simulink model "TSR_Scenario_002_cs"  (Simulink 7.6)
settings: Solver=ode5  SolverMode=SingleTasking  StartTime=0.0  StopTime=inf  FixedStep=1/100  RelTol=1e-3
PreLoadFcn:
  | check_NUTS_defined;
  | 
  | assignin('base',['model_closed_' bdroot],false);
  | 
  | addpath('../includes_TSR/includes_TSR');
  | 
  | switch version('-release')
  | case {'2010a', '2010b', '2011a'}
  | addpath('../includes_TSR/ML2010b');
  | otherwise
  | addpath('../includes_TSR/ML2011b');
  | end
InitFcn:
  | PreScanExperimentInitFcn(bdroot);
  | 
  | addpath('..\includes_TSR');
StopFcn:
  | PreScanExperimentStopFcn(bdroot)
undefined symbols (not defined in the file): DiscIntNeverNeededParam, InitImageOutputDir, x_ini_VRML, y_ini_VRML, z_ini_VRML
libraries: PreScanSimulinkLibrary x79, simulink x28, PreScanUsefulBlocks x21, simulink_extras x12, simulink_need_slupdate x1
(part 3 of 36)

== system TSR_Scenario_002_cs/Ford_Fiesta_1 ==
  SELF_Ford_Fiesta_1 : Inport  Tag=SELFInport
  SELF_Demux : Subsystem  Tag=DemuxSELF
      settings: DeleteFcn=PreScanBlockDeleteFcn;
      mask Type: PreScan Demux Block:
      mask Display:
      | port_label('output',1,'x [m]')
      | port_label('output',2,'y [m]')
      | port_label('output',3,'z [m]','texmode','on')
      | port_label('output',4,'Rotx [deg]','texmode','on')
      | port_label('output',5,'Roty [deg]','texmode','on')
      | port_label('output',6,'Rotz [deg]','texmode','on')
      | port_label('output',7,'GPS lat [deg]','texmode','on')
      | port_label('output',8,'GPS long [deg]','texmode','on')
      | port_label('output',9,'GPS alt [m]','texmode','on')
      | port_label('output',10,'Velocity [m/s]')
      | port_label('output',11,'Heading [deg]')
      | port_label('output',12,'Yaw rate [deg/s]')
  Terminator_{1..12} (12 alike) : Terminator
  Trajectories : Subsystem  Tag=Trajectories
      settings: DeleteFcn=PreScanBlockDeleteFcn;
      mask Type: PreScan Source Block: Trajectories
      mask Description:
      | The Trajectory block contains the trajectories defined in the PreScan Experiment Editor. The output of the trajectory block is the (vehicle) State.
      | 
      | For more information, please click Help.
      mask Help: eval('openHelp(312)')
      mask Display: image(imread('Trajectory.png'));
  STATE_Ford_Fiesta_1 : Outport  Tag=StateOutport
  wires:
  SELF_Ford_Fiesta_1/1 -> SELF_Demux/1(SELF)
  Trajectories/1(State) -> STATE_Ford_Fiesta_1/1
  SELF_Demux/1(x [m]) -> Terminator_1/1
  SELF_Demux/2(y [m]) -> Terminator_2/1
  SELF_Demux/3(z [m]) -> Terminator_3/1
  SELF_Demux/4(rotx [deg]) -> Terminator_4/1
  SELF_Demux/5(roty [deg]) -> Terminator_5/1
  SELF_Demux/6(rotz [deg]) -> Terminator_6/1
  SELF_Demux/7(GPS lat [deg]) -> Terminator_7/1
  SELF_Demux/8(GPS long [deg]) -> Terminator_8/1
  SELF_Demux/9(GPS alt [m]) -> Terminator_9/1
  SELF_Demux/10(velocity [m/s]) -> Terminator_10/1
  SELF_Demux/11(heading [deg]) -> Terminator_11/1
  SELF_Demux/12(yaw rate [deg/s]) -> Terminator_12/1

== system TSR_Scenario_002_cs/GetSensors ==
  dummy : Inport
  GetSensors : M-S-Function  FunctionName=GetSensorsBlock  Parameters=TimeStep NrOfSensors
  tag scope: SimCoreSensors=global
  wires:
  GetSensors/1 -> [SimCoreSensors]
  dummy/1 -> GetSensors/1

== system TSR_Scenario_002_cs/HighwayOverhang_1 ==
  note: Resources
      |
      | 1: InformationSign\MatrixSign_GreenArrow.png: MatrixSign GreenArrow
      | 2: InformationSign\TASS_logo.jpeg: TASS logo
      | 3: InformationSign\SEARCH_Logo.png: SEARCH Logo
      | 4: InformationSign\z274-50_black_full.png: z274-50 black full
      | 5: InformationSign\z274-51_black_full.png: z274-51 black full
      | 6: InformationSign\z274-52_black_full.png: z274-52 black full
      | 7: InformationSign\z274-53_black_full.png: z274-53 black full
      | 8: InformationSign\z274-54_black_full.png: z274-54 black full
      | 9: InformationSign\z274-55_black_full.png: z274-55 black full
      | 10: InformationSign\z274-56_black_full.png: z274-56 black full
      | 11: InformationSign\z274-57_black_full.png: z274-57 black full
      | 12: InformationSign\z274-58_black_full.png: z274-58 black full
      | 13: InformationSign\z274-59_black_full.png: z274-59 black full
      | 14: InformationSign\z274-60_black_full.png: z274-60 black full
      | 15: InformationSign\z274-61_black_full.png: z274-61 black full
      | 16: InformationSign\z274-62_black_full.png: z274-62 black full
      | 17: InformationSign\z274-63_black_full.png: z274-63 black full
  note: Resources
  Ground_{1..4} (4 alike) : Ground
  VISUMatrixSignLights_Mux : Subsystem  Tag=MuxMatrixSignLights
      settings: DeleteFcn=PreScanBlockDeleteFcn;
      mask Type: PreScan Mux Block:
  VISUMatrixSign_Mux : Subsystem  Tag=MuxMatrixSign
      settings: DeleteFcn=PreScanBlockDeleteFcn;
      mask Type: PreScan Mux Block:
  VISUMatrixSign_HighwayOverhang_1 : Outport  Tag=VisuMatrixSignOutport  SampleTime=1/100
  VISUMatrixSignLights_HighwayOverhang_1 : Outport  Tag=VisuMatrixSignLightsOutport  Port=2  SampleTime=1/100
  wires:
  VISUMatrixSign_Mux/1(MatrixSigns) -> VISUMatrixSign_HighwayOverhang_1/1
  VISUMatrixSignLights_Mux/1(MatrixSignLights) -> VISUMatrixSignLights_HighwayOverhang_1/1
  Ground_1/1 -> VISUMatrixSignLights_Mux/1(MatrixSign_1)
  Ground_2/1 -> VISUMatrixSignLights_Mux/2(MatrixSign_43)
  Ground_3/1 -> VISUMatrixSign_Mux/1(MatrixSign_1)
  Ground_4/1 -> VISUMatrixSign_Mux/2(MatrixSign_43)

== system TSR_Scenario_002_cs/HighwayOverhang_10 ==
  note: Resources
      |
      | 1: InformationSign\MatrixSign_GreenArrow.png: MatrixSign GreenArrow
      | 2: InformationSign\TASS_logo.jpeg: TASS logo
      | 3: InformationSign\SEARCH_Logo.png: SEARCH Logo
      | 4: InformationSign\z274-50_black_full.png: z274-50 black full
      | 5: InformationSign\z274-51_black_full.png: z274-51 black full
      | 6: InformationSign\z274-52_black_full.png: z274-52 black full
      | 7: InformationSign\z274-53_black_full.png: z274-53 black full
      | 8: InformationSign\z274-54_black_full.png: z274-54 black full
      | 9: InformationSign\z274-55_black_full.png: z274-55 black full
      | 10: InformationSign\z274-56_black_full.png: z274-56 black full
      | 11: InformationSign\z274-57_black_full.png: z274-57 black full
      | 12: InformationSign\z274-58_black_full.png: z274-58 black full
      | 13: InformationSign\z274-59_black_full.png: z274-59 black full
      | 14: InformationSign\z274-60_black_full.png: z274-60 black full
      | 15: InformationSign\z274-61_black_full.png: z274-61 black full
      | 16: InformationSign\z274-62_black_full.png: z274-62 black full
      | 17: InformationSign\z274-63_black_full.png: z274-63 black full
  note: Resources
  Ground_{1..4} (4 alike) : Ground
  VISUMatrixSignLights_Mux : Subsystem  Tag=MuxMatrixSignLights
      settings: DeleteFcn=PreScanBlockDeleteFcn;
      mask Type: PreScan Mux Block:
  VISUMatrixSign_Mux : Subsystem  Tag=MuxMatrixSign
      settings: DeleteFcn=PreScanBlockDeleteFcn;
      mask Type: PreScan Mux Block:
  VISUMatrixSign_HighwayOverhang_10 : Outport  Tag=VisuMatrixSignOutport  SampleTime=1/100
  VISUMatrixSignLights_HighwayOverhang_10 : Outport  Tag=VisuMatrixSignLightsOutport  Port=2  SampleTime=1/100
  wires:
  VISUMatrixSign_Mux/1(MatrixSigns) -> VISUMatrixSign_HighwayOverhang_10/1
  VISUMatrixSignLights_Mux/1(MatrixSignLights) -> VISUMatrixSignLights_HighwayOverhang_10/1
  Ground_1/1 -> VISUMatrixSignLights_Mux/1(MatrixSign_17)
  Ground_2/1 -> VISUMatrixSignLights_Mux/2(MatrixSign_18)
  Ground_3/1 -> VISUMatrixSign_Mux/1(MatrixSign_17)
  Ground_4/1 -> VISUMatrixSign_Mux/2(MatrixSign_18)

== system TSR_Scenario_002_cs/HighwayOverhang_11 ==
  note: Resources
      |
      | 1: InformationSign\MatrixSign_GreenArrow.png: MatrixSign GreenArrow
      | 2: InformationSign\TASS_logo.jpeg: TASS logo
      | 3: InformationSign\SEARCH_Logo.png: SEARCH Logo
      | 4: InformationSign\z274-50_black_full.png: z274-50 black full
      | 5: InformationSign\z274-51_black_full.png: z274-51 black full
      | 6: InformationSign\z274-52_black_full.png: z274-52 black full
      | 7: InformationSign\z274-53_black_full.png: z274-53 black full
      | 8: InformationSign\z274-54_black_full.png: z274-54 black full
      | 9: InformationSign\z274-55_black_full.png: z274-55 black full
      | 10: InformationSign\z274-56_black_full.png: z274-56 black full
      | 11: InformationSign\z274-57_black_full.png: z274-57 black full
      | 12: InformationSign\z274-58_black_full.png: z274-58 black full
      | 13: InformationSign\z274-59_black_full.png: z274-59 black full
      | 14: InformationSign\z274-60_black_full.png: z274-60 black full
      | 15: InformationSign\z274-61_black_full.png: z274-61 black full
      | 16: InformationSign\z274-62_black_full.png: z274-62 black full
      | 17: InformationSign\z274-63_black_full.png: z274-63 black full
  note: Resources
  Ground_{1..4} (4 alike) : Ground
  VISUMatrixSignLights_Mux : Subsystem  Tag=MuxMatrixSignLights
      settings: DeleteFcn=PreScanBlockDeleteFcn;
      mask Type: PreScan Mux Block:
  VISUMatrixSign_Mux : Subsystem  Tag=MuxMatrixSign
      settings: DeleteFcn=PreScanBlockDeleteFcn;
      mask Type: PreScan Mux Block:
  VISUMatrixSign_HighwayOverhang_11 : Outport  Tag=VisuMatrixSignOutport  SampleTime=1/100
  VISUMatrixSignLights_HighwayOverhang_11 : Outport  Tag=VisuMatrixSignLightsOutport  Port=2  SampleTime=1/100
  wires:
  VISUMatrixSign_Mux/1(MatrixSigns) -> VISUMatrixSign_HighwayOverhang_11/1
  VISUMatrixSignLights_Mux/1(MatrixSignLights) -> VISUMatrixSignLights_HighwayOverhang_11/1
  Ground_1/1 -> VISUMatrixSignLights_Mux/1(MatrixSign_10)
  Ground_2/1 -> VISUMatrixSignLights_Mux/2(MatrixSign_49)
  Ground_3/1 -> VISUMatrixSign_Mux/1(MatrixSign_10)
  Ground_4/1 -> VISUMatrixSign_Mux/2(MatrixSign_49)

== system TSR_Scenario_002_cs/HighwayOverhang_12 ==
  note: Resources
      |
      | 1: InformationSign\MatrixSign_GreenArrow.png: MatrixSign GreenArrow
      | 2: InformationSign\TASS_logo.jpeg: TASS logo
      | 3: InformationSign\SEARCH_Logo.png: SEARCH Logo
      | 4: InformationSign\z274-50_black_full.png: z274-50 black full
      | 5: InformationSign\z274-51_black_full.png: z274-51 black full
      | 6: InformationSign\z274-52_black_full.png: z274-52 black full
      | 7: InformationSign\z274-53_black_full.png: z274-53 black full
      | 8: InformationSign\z274-54_black_full.png: z274-54 black full
      | 9: InformationSign\z274-55_black_full.png: z274-55 black full
      | 10: InformationSign\z274-56_black_full.png: z274-56 black full
      | 11: InformationSign\z274-57_black_full.png: z274-57 black full
      | 12: InformationSign\z274-58_black_full.png: z274-58 black full
      | 13: InformationSign\z274-59_black_full.png: z274-59 black full
      | 14: InformationSign\z274-60_black_full.png: z274-60 black full
      | 15: InformationSign\z274-61_black_full.png: z274-61 black full
      | 16: InformationSign\z274-62_black_full.png: z274-62 black full
      | 17: InformationSign\z274-63_black_full.png: z274-63 black full
  note: Resources
  Ground_{1..4} (4 alike) : Ground
  VISUMatrixSignLights_Mux : Subsystem  Tag=MuxMatrixSignLights
      settings: DeleteFcn=PreScanBlockDeleteFcn;
      mask Type: PreScan Mux Block:
  VISUMatrixSign_Mux : Subsystem  Tag=MuxMatrixSign
      settings: DeleteFcn=PreScanBlockDeleteFcn;
      mask Type: PreScan Mux Block:
  VISUMatrixSign_HighwayOverhang_12 : Outport  Tag=VisuMatrixSignOutport  SampleTime=1/100
  VISUMatrixSignLights_HighwayOverhang_12 : Outport  Tag=VisuMatrixSignLightsOutport  Port=2  SampleTime=1/100
  wires:
  VISUMatrixSign_Mux/1(MatrixSigns) -> VISUMatrixSign_HighwayOverhang_12/1
  VISUMatrixSignLights_Mux/1(MatrixSignLights) -> VISUMatrixSignLights_HighwayOverhang_12/1
  Ground_1/1 -> VISUMatrixSignLights_Mux/1(MatrixSign_21)
  Ground_2/1 -> VISUMatrixSignLights_Mux/2(MatrixSign_22)
  Ground_3/1 -> VISUMatrixSign_Mux/1(MatrixSign_21)
  Ground_4/1 -> VISUMatrixSign_Mux/2(MatrixSign_22)

== system TSR_Scenario_002_cs/HighwayOverhang_13 ==
  note: Resources
      |
      | 1: InformationSign\MatrixSign_GreenArrow.png: MatrixSign GreenArrow
      | 2: InformationSign\TASS_logo.jpeg: TASS logo
      | 3: InformationSign\SEARCH_Logo.png: SEARCH Logo
      | 4: InformationSign\z274-50_black_full.png: z274-50 black full
      | 5: InformationSign\z274-51_black_full.png: z274-51 black full
      | 6: InformationSign\z274-52_black_full.png: z274-52 black full
      | 7: InformationSign\z274-53_black_full.png: z274-53 black full
      | 8: InformationSign\z274-54_black_full.png: z274-54 black full
      | 9: InformationSign\z274-55_black_full.png: z274-55 black full
      | 10: InformationSign\z274-56_black_full.png: z274-56 black full
      | 11: InformationSign\z274-57_black_full.png: z274-57 black full
      | 12: InformationSign\z274-58_black_full.png: z274-58 black full
      | 13: InformationSign\z274-59_black_full.png: z274-59 black full
      | 14: InformationSign\z274-60_black_full.png: z274-60 black full
      | 15: InformationSign\z274-61_black_full.png: z274-61 black full
      | 16: InformationSign\z274-62_black_full.png: z274-62 black full
      | 17: InformationSign\z274-63_black_full.png: z274-63 black full
  note: Resources
  Ground_{1..4} (4 alike) : Ground
  VISUMatrixSignLights_Mux : Subsystem  Tag=MuxMatrixSignLights
      settings: DeleteFcn=PreScanBlockDeleteFcn;
      mask Type: PreScan Mux Block:
  VISUMatrixSign_Mux : Subsystem  Tag=MuxMatrixSign
      settings: DeleteFcn=PreScanBlockDeleteFcn;
      mask Type: PreScan Mux Block:
  VISUMatrixSign_HighwayOverhang_13 : Outport  Tag=VisuMatrixSignOutport  SampleTime=1/100
  VISUMatrixSignLights_HighwayOverhang_13 : Outport  Tag=VisuMatrixSignLightsOutport  Port=2  SampleTime=1/100
  wires:
  VISUMatrixSign_Mux/1(MatrixSigns) -> VISUMatrixSign_HighwayOverhang_13/1
  VISUMatrixSignLights_Mux/1(MatrixSignLights) -> VISUMatrixSignLights_HighwayOverhang_13/1
  Ground_1/1 -> VISUMatrixSignLights_Mux/1(MatrixSign_3)
  Ground_2/1 -> VISUMatrixSignLights_Mux/2(MatrixSign_44)
  Ground_3/1 -> VISUMatrixSign_Mux/1(MatrixSign_3)
  Ground_4/1 -> VISUMatrixSign_Mux/2(MatrixSign_44)

== system TSR_Scenario_002_cs/HighwayOverhang_14 ==
  note: Resources
      |
      | 1: InformationSign\MatrixSign_GreenArrow.png: MatrixSign GreenArrow
      | 2: InformationSign\TASS_logo.jpeg: TASS logo
      | 3: InformationSign\SEARCH_Logo.png: SEARCH Logo
      | 4: InformationSign\z274-50_black_full.png: z274-50 black full
      | 5: InformationSign\z274-51_black_full.png: z274-51 black full
      | 6: InformationSign\z274-52_black_full.png: z274-52 black full
      | 7: InformationSign\z274-53_black_full.png: z274-53 black full
      | 8: InformationSign\z274-54_black_full.png: z274-54 black full
      | 9: InformationSign\z274-55_black_full.png: z274-55 black full
      | 10: InformationSign\z274-56_black_full.png: z274-56 black full
      | 11: InformationSign\z274-57_black_full.png: z274-57 black full
      | 12: InformationSign\z274-58_black_full.png: z274-58 black full
      | 13: InformationSign\z274-59_black_full.png: z274-59 black full
      | 14: InformationSign\z274-60_black_full.png: z274-60 black full
      | 15: InformationSign\z274-61_black_full.png: z274-61 black full
      | 16: InformationSign\z274-62_black_full.png: z274-62 black full
      | 17: InformationSign\z274-63_black_full.png: z274-63 black full
  note: Resources
  Ground_{1..4} (4 alike) : Ground
  VISUMatrixSignLights_Mux : Subsystem  Tag=MuxMatrixSignLights
      settings: DeleteFcn=PreScanBlockDeleteFcn;
      mask Type: PreScan Mux Block:
  VISUMatrixSign_Mux : Subsystem  Tag=MuxMatrixSign
      settings: DeleteFcn=PreScanBlockDeleteFcn;
      mask Type: PreScan Mux Block:
  VISUMatrixSign_HighwayOverhang_14 : Outport  Tag=VisuMatrixSignOutport  SampleTime=1/100
  VISUMatrixSignLights_HighwayOverhang_14 : Outport  Tag=VisuMatrixSignLightsOutport  Port=2  SampleTime=1/100
  wires:
  VISUMatrixSign_Mux/1(MatrixSigns) -> VISUMatrixSign_HighwayOverhang_14/1
  VISUMatrixSignLights_Mux/1(MatrixSignLights) -> VISUMatrixSignLights_HighwayOverhang_14/1
  Ground_1/1 -> VISUMatrixSignLights_Mux/1(MatrixSign_27)
  Ground_2/1 -> VISUMatrixSignLights_Mux/2(MatrixSign_28)
  Ground_3/1 -> VISUMatrixSign_Mux/1(MatrixSign_27)
  Ground_4/1 -> VISUMatrixSign_Mux/2(MatrixSign_28)

== system TSR_Scenario_002_cs/HighwayOverhang_15 ==
  note: Resources
      |
      | 1: InformationSign\MatrixSign_GreenArrow.png: MatrixSign GreenArrow
      | 2: InformationSign\TASS_logo.jpeg: TASS logo
      | 3: InformationSign\SEARCH_Logo.png: SEARCH Logo
      | 4: InformationSign\z274-50_black_full.png: z274-50 black full
      | 5: InformationSign\z274-51_black_full.png: z274-51 black full
      | 6: InformationSign\z274-52_black_full.png: z274-52 black full
      | 7: InformationSign\z274-53_black_full.png: z274-53 black full
      | 8: InformationSign\z274-54_black_full.png: z274-54 black full
      | 9: InformationSign\z274-55_black_full.png: z274-55 black full
      | 10: InformationSign\z274-56_black_full.png: z274-56 black full
      | 11: InformationSign\z274-57_black_full.png: z274-57 black full
      | 12: InformationSign\z274-58_black_full.png: z274-58 black full
      | 13: InformationSign\z274-59_black_full.png: z274-59 black full
      | 14: InformationSign\z274-60_black_full.png: z274-60 black full
      | 15: InformationSign\z274-61_black_full.png: z274-61 black full
      | 16: InformationSign\z274-62_black_full.png: z274-62 black full
      | 17: InformationSign\z274-63_black_full.png: z274-63 black full
  note: Resources
  Ground_{1..4} (4 alike) : Ground
  VISUMatrixSignLights_Mux : Subsystem  Tag=MuxMatrixSignLights
      settings: DeleteFcn=PreScanBlockDeleteFcn;
      mask Type: PreScan Mux Block:
  VISUMatrixSign_Mux : Subsystem  Tag=MuxMatrixSign
      settings: DeleteFcn=PreScanBlockDeleteFcn;
      mask Type: PreScan Mux Block:
  VISUMatrixSign_HighwayOverhang_15 : Outport  Tag=VisuMatrixSignOutport  SampleTime=1/100
  VISUMatrixSignLights_HighwayOverhang_15 : Outport  Tag=VisuMatrixSignLightsOutport  Port=2  SampleTime=1/100
  wires:
  VISUMatrixSign_Mux/1(MatrixSigns) -> VISUMatrixSign_HighwayOverhang_15/1
  VISUMatrixSignLights_Mux/1(MatrixSignLights) -> VISUMatrixSignLights_HighwayOverhang_15/1
  Ground_1/1 -> VISUMatrixSignLights_Mux/1(MatrixSign_5)
  Ground_2/1 -> VISUMatrixSignLights_Mux/2(MatrixSign_46)
  Ground_3/1 -> VISUMatrixSign_Mux/1(MatrixSign_5)
  Ground_4/1 -> VISUMatrixSign_Mux/2(MatrixSign_46)

== system TSR_Scenario_002_cs/HighwayOverhang_16 ==
  note: Resources
      |
      | 1: InformationSign\MatrixSign_GreenArrow.png: MatrixSign GreenArrow
      | 2: InformationSign\TASS_logo.jpeg: TASS logo
      | 3: InformationSign\SEARCH_Logo.png: SEARCH Logo
      | 4: InformationSign\z274-50_black_full.png: z274-50 black full
      | 5: InformationSign\z274-51_black_full.png: z274-51 black full
      | 6: InformationSign\z274-52_black_full.png: z274-52 black full
      | 7: InformationSign\z274-53_black_full.png: z274-53 black full
      | 8: InformationSign\z274-54_black_full.png: z274-54 black full
      | 9: InformationSign\z274-55_black_full.png: z274-55 black full
      | 10: InformationSign\z274-56_black_full.png: z274-56 black full
      | 11: InformationSign\z274-57_black_full.png: z274-57 black full
      | 12: InformationSign\z274-58_black_full.png: z274-58 black full
      | 13: InformationSign\z274-59_black_full.png: z274-59 black full
      | 14: InformationSign\z274-60_black_full.png: z274-60 black full
      | 15: InformationSign\z274-61_black_full.png: z274-61 black full
      | 16: InformationSign\z274-62_black_full.png: z274-62 black full
      | 17: InformationSign\z274-63_black_full.png: z274-63 black full
  note: Resources
  Ground_{1..4} (4 alike) : Ground
  VISUMatrixSignLights_Mux : Subsystem  Tag=MuxMatrixSignLights
      settings: DeleteFcn=PreScanBlockDeleteFcn;
      mask Type: PreScan Mux Block:
  VISUMatrixSign_Mux : Subsystem  Tag=MuxMatrixSign
      settings: DeleteFcn=PreScanBlockDeleteFcn;
      mask Type: PreScan Mux Block:
  VISUMatrixSign_HighwayOverhang_16 : Outport  Tag=VisuMatrixSignOutport  SampleTime=1/100
  VISUMatrixSignLights_HighwayOverhang_16 : Outport  Tag=VisuMatrixSignLightsOutport  Port=2  SampleTime=1/100
  wires:
  VISUMatrixSign_Mux/1(MatrixSigns) -> VISUMatrixSign_HighwayOverhang_16/1
  VISUMatrixSignLights_Mux/1(MatrixSignLights) -> VISUMatrixSignLights_HighwayOverhang_16/1
  Ground_1/1 -> VISUMatrixSignLights_Mux/1(MatrixSign_29)
  Ground_2/1 -> VISUMatrixSignLights_Mux/2(MatrixSign_30)
  Ground_3/1 -> VISUMatrixSign_Mux/1(MatrixSign_29)
  Ground_4/1 -> VISUMatrixSign_Mux/2(MatrixSign_30)

== system TSR_Scenario_002_cs/HighwayOverhang_17 ==
  note: Resources
      |
      | 1: InformationSign\MatrixSign_GreenArrow.png: MatrixSign GreenArrow
      | 2: InformationSign\TASS_logo.jpeg: TASS logo
      | 3: InformationSign\SEARCH_Logo.png: SEARCH Logo
      | 4: InformationSign\z274-50_black_full.png: z274-50 black full
      | 5: InformationSign\z274-51_black_full.png: z274-51 black full
      | 6: InformationSign\z274-52_black_full.png: z274-52 black full
      | 7: InformationSign\z274-53_black_full.png: z274-53 black full
      | 8: InformationSign\z274-54_black_full.png: z274-54 black full
      | 9: InformationSign\z274-55_black_full.png: z274-55 black full
      | 10: InformationSign\z274-56_black_full.png: z274-56 black full
      | 11: InformationSign\z274-57_black_full.png: z274-57 black full
      | 12: InformationSign\z274-58_black_full.png: z274-58 black full
      | 13: InformationSign\z274-59_black_full.png: z274-59 black full
      | 14: InformationSign\z274-60_black_full.png: z274-60 black full
      | 15: InformationSign\z274-61_black_full.png: z274-61 black full
      | 16: InformationSign\z274-62_black_full.png: z274-62 black full
      | 17: InformationSign\z274-63_black_full.png: z274-63 black full
  note: Resources
  Ground_{1..4} (4 alike) : Ground
  VISUMatrixSignLights_Mux : Subsystem  Tag=MuxMatrixSignLights
      settings: DeleteFcn=PreScanBlockDeleteFcn;
      mask Type: PreScan Mux Block:
  VISUMatrixSign_Mux : Subsystem  Tag=MuxMatrixSign
      settings: DeleteFcn=PreScanBlockDeleteFcn;
      mask Type: PreScan Mux Block:
  VISUMatrixSign_HighwayOverhang_17 : Outport  Tag=VisuMatrixSignOutport  SampleTime=1/100
  VISUMatrixSignLights_HighwayOverhang_17 : Outport  Tag=VisuMatrixSignLightsOutport  Port=2  SampleTime=1/100
  wires:
  VISUMatrixSign_Mux/1(MatrixSigns) -> VISUMatrixSign_HighwayOverhang_17/1
  VISUMatrixSignLights_Mux/1(MatrixSignLights) -> VISUMatrixSignLights_HighwayOverhang_17/1
  Ground_1/1 -> VISUMatrixSignLights_Mux/1(MatrixSign_7)
  Ground_2/1 -> VISUMatrixSignLights_Mux/2(MatrixSign_51)
  Ground_3/1 -> VISUMatrixSign_Mux/1(MatrixSign_7)
  Ground_4/1 -> VISUMatrixSign_Mux/2(MatrixSign_51)

== system TSR_Scenario_002_cs/HighwayOverhang_18 ==
  note: Resources
      |
      | 1: InformationSign\MatrixSign_GreenArrow.png: MatrixSign GreenArrow
      | 2: InformationSign\TASS_logo.jpeg: TASS logo
      | 3: InformationSign\SEARCH_Logo.png: SEARCH Logo
      | 4: InformationSign\z274-50_black_full.png: z274-50 black full
      | 5: InformationSign\z274-51_black_full.png: z274-51 black full
      | 6: InformationSign\z274-52_black_full.png: z274-52 black full
      | 7: InformationSign\z274-53_black_full.png: z274-53 black full
      | 8: InformationSign\z274-54_black_full.png: z274-54 black full
      | 9: InformationSign\z274-55_black_full.png: z274-55 black full
      | 10: InformationSign\z274-56_black_full.png: z274-56 black full
      | 11: InformationSign\z274-57_black_full.png: z274-57 black full
      | 12: InformationSign\z274-58_black_full.png: z274-58 black full
      | 13: InformationSign\z274-59_black_full.png: z274-59 black full
      | 14: InformationSign\z274-60_black_full.png: z274-60 black full
      | 15: InformationSign\z274-61_black_full.png: z274-61 black full
      | 16: InformationSign\z274-62_black_full.png: z274-62 black full
      | 17: InformationSign\z274-63_black_full.png: z274-63 black full
  note: Resources
  Ground_{1..4} (4 alike) : Ground
  VISUMatrixSignLights_Mux : Subsystem  Tag=MuxMatrixSignLights
      settings: DeleteFcn=PreScanBlockDeleteFcn;
      mask Type: PreScan Mux Block:
  VISUMatrixSign_Mux : Subsystem  Tag=MuxMatrixSign
      settings: DeleteFcn=PreScanBlockDeleteFcn;
      mask Type: PreScan Mux Block:
  VISUMatrixSign_HighwayOverhang_18 : Outport  Tag=VisuMatrixSignOutport  SampleTime=1/100
  VISUMatrixSignLights_HighwayOverhang_18 : Outport  Tag=VisuMatrixSignLightsOutport  Port=2  SampleTime=1/100
  wires:
  VISUMatrixSign_Mux/1(MatrixSigns) -> VISUMatrixSign_HighwayOverhang_18/1
  VISUMatrixSignLights_Mux/1(MatrixSignLights) -> VISUMatrixSignLights_HighwayOverhang_18/1
  Ground_1/1 -> VISUMatrixSignLights_Mux/1(MatrixSign_31)
  Ground_2/1 -> VISUMatrixSignLights_Mux/2(MatrixSign_32)
  Ground_3/1 -> VISUMatrixSign_Mux/1(MatrixSign_31)
  Ground_4/1 -> VISUMatrixSign_Mux/2(MatrixSign_32)

== system TSR_Scenario_002_cs/HighwayOverhang_19 ==
  note: Resources
      |
      | 1: InformationSign\MatrixSign_GreenArrow.png: MatrixSign GreenArrow
      | 2: InformationSign\TASS_logo.jpeg: TASS logo
      | 3: InformationSign\SEARCH_Logo.png: SEARCH Logo
      | 4: InformationSign\z274-50_black_full.png: z274-50 black full
      | 5: InformationSign\z274-51_black_full.png: z274-51 black full
      | 6: InformationSign\z274-52_black_full.png: z274-52 black full
      | 7: InformationSign\z274-53_black_full.png: z274-53 black full
      | 8: InformationSign\z274-54_black_full.png: z274-54 black full
      | 9: InformationSign\z274-55_black_full.png: z274-55 black full
      | 10: InformationSign\z274-56_black_full.png: z274-56 black full
      | 11: InformationSign\z274-57_black_full.png: z274-57 black full
      | 12: InformationSign\z274-58_black_full.png: z274-58 black full
      | 13: InformationSign\z274-59_black_full.png: z274-59 black full
      | 14: InformationSign\z274-60_black_full.png: z274-60 black full
      | 15: InformationSign\z274-61_black_full.png: z274-61 black full
      | 16: InformationSign\z274-62_black_full.png: z274-62 black full
      | 17: InformationSign\z274-63_black_full.png: z274-63 black full
  note: Resources
  Ground_{1..4} (4 alike) : Ground
  VISUMatrixSignLights_Mux : Subsystem  Tag=MuxMatrixSignLights
      settings: DeleteFcn=PreScanBlockDeleteFcn;
      mask Type: PreScan Mux Block:
  VISUMatrixSign_Mux : Subsystem  Tag=MuxMatrixSign
      settings: DeleteFcn=PreScanBlockDeleteFcn;
      mask Type: PreScan Mux Block:
  VISUMatrixSign_HighwayOverhang_19 : Outport  Tag=VisuMatrixSignOutport  SampleTime=1/100
  VISUMatrixSignLights_HighwayOverhang_19 : Outport  Tag=VisuMatrixSignLightsOutport  Port=2  SampleTime=1/100
  wires:
  VISUMatrixSign_Mux/1(MatrixSigns) -> VISUMatrixSign_HighwayOverhang_19/1
  VISUMatrixSignLights_Mux/1(MatrixSignLights) -> VISUMatrixSignLights_HighwayOverhang_19/1
  Ground_1/1 -> VISUMatrixSignLights_Mux/1(MatrixSign_9)
  Ground_2/1 -> VISUMatrixSignLights_Mux/2(MatrixSign_52)
  Ground_3/1 -> VISUMatrixSign_Mux/1(MatrixSign_9)
  Ground_4/1 -> VISUMatrixSign_Mux/2(MatrixSign_52)

== system TSR_Scenario_002_cs/HighwayOverhang_2 ==
  note: Resources
      |
      | 1: InformationSign\MatrixSign_GreenArrow.png: MatrixSign GreenArrow
      | 2: InformationSign\TASS_logo.jpeg: TASS logo
      | 3: InformationSign\SEARCH_Logo.png: SEARCH Logo
      | 4: InformationSign\z274-50_black_full.png: z274-50 black full
      | 5: InformationSign\z274-51_black_full.png: z274-51 black full
      | 6: InformationSign\z274-52_black_full.png: z274-52 black full
      | 7: InformationSign\z274-53_black_full.png: z274-53 black full
      | 8: InformationSign\z274-54_black_full.png: z274-54 black full
      | 9: InformationSign\z274-55_black_full.png: z274-55 black full
      | 10: InformationSign\z274-56_black_full.png: z274-56 black full
      | 11: InformationSign\z274-57_black_full.png: z274-57 black full
      | 12: InformationSign\z274-58_black_full.png: z274-58 black full
      | 13: InformationSign\z274-59_black_full.png: z274-59 black full
      | 14: InformationSign\z274-60_black_full.png: z274-60 black full
      | 15: InformationSign\z274-61_black_full.png: z274-61 black full
      | 16: InformationSign\z274-62_black_full.png: z274-62 black full
      | 17: InformationSign\z274-63_black_full.png: z274-63 black full
  note: Resources
  Ground_{1..4} (4 alike) : Ground
  VISUMatrixSignLights_Mux : Subsystem  Tag=MuxMatrixSignLights
      settings: DeleteFcn=PreScanBlockDeleteFcn;
      mask Type: PreScan Mux Block:
  VISUMatrixSign_Mux : Subsystem  Tag=MuxMatrixSign
      settings: DeleteFcn=PreScanBlockDeleteFcn;
      mask Type: PreScan Mux Block:
  VISUMatrixSign_HighwayOverhang_2 : Outport  Tag=VisuMatrixSignOutport  SampleTime=1/100
  VISUMatrixSignLights_HighwayOverhang_2 : Outport  Tag=VisuMatrixSignLightsOutport  Port=2  SampleTime=1/100
  wires:
  VISUMatrixSign_Mux/1(MatrixSigns) -> VISUMatrixSign_HighwayOverhang_2/1
  VISUMatrixSignLights_Mux/1(MatrixSignLights) -> VISUMatrixSignLights_HighwayOverhang_2/1
  Ground_1/1 -> VISUMatrixSignLights_Mux/1(MatrixSign_14)
  Ground_2/1 -> VISUMatrixSignLights_Mux/2(MatrixSign_2)
  Ground_3/1 -> VISUMatrixSign_Mux/1(MatrixSign_14)
  Ground_4/1 -> VISUMatrixSign_Mux/2(MatrixSign_2)

== system TSR_Scenario_002_cs/HighwayOverhang_20 ==
  note: Resources
      |
      | 1: InformationSign\MatrixSign_GreenArrow.png: MatrixSign GreenArrow
      | 2: InformationSign\TASS_logo.jpeg: TASS logo
      | 3: InformationSign\SEARCH_Logo.png: SEARCH Logo
      | 4: InformationSign\z274-50_black_full.png: z274-50 black full
      | 5: InformationSign\z274-51_black_full.png: z274-51 black full
      | 6: InformationSign\z274-52_black_full.png: z274-52 black full
      | 7: InformationSign\z274-53_black_full.png: z274-53 black full
      | 8: InformationSign\z274-54_black_full.png: z274-54 black full
      | 9: InformationSign\z274-55_black_full.png: z274-55 black full
      | 10: InformationSign\z274-56_black_full.png: z274-56 black full
      | 11: InformationSign\z274-57_black_full.png: z274-57 black full
      | 12: InformationSign\z274-58_black_full.png: z274-58 black full
      | 13: InformationSign\z274-59_black_full.png: z274-59 black full
      | 14: InformationSign\z274-60_black_full.png: z274-60 black full
      | 15: InformationSign\z274-61_black_full.png: z274-61 black full
      | 16: InformationSign\z274-62_black_full.png: z274-62 black full
      | 17: InformationSign\z274-63_black_full.png: z274-63 black full
  note: Resources
  Ground_{1..4} (4 alike) : Ground
  VISUMatrixSignLights_Mux : Subsystem  Tag=MuxMatrixSignLights
      settings: DeleteFcn=PreScanBlockDeleteFcn;
      mask Type: PreScan Mux Block:
  VISUMatrixSign_Mux : Subsystem  Tag=MuxMatrixSign
      settings: DeleteFcn=PreScanBlockDeleteFcn;
      mask Type: PreScan Mux Block:
  VISUMatrixSign_HighwayOverhang_20 : Outport  Tag=VisuMatrixSignOutport  SampleTime=1/100
  VISUMatrixSignLights_HighwayOverhang_20 : Outport  Tag=VisuMatrixSignLightsOutport  Port=2  SampleTime=1/100
  wires:
  VISUMatrixSign_Mux/1(MatrixSigns) -> VISUMatrixSign_HighwayOverhang_20/1
  VISUMatrixSignLights_Mux/1(MatrixSignLights) -> VISUMatrixSignLights_HighwayOverhang_20/1
  Ground_1/1 -> VISUMatrixSignLights_Mux/1(MatrixSign_33)
  Ground_2/1 -> VISUMatrixSignLights_Mux/2(MatrixSign_34)
  Ground_3/1 -> VISUMatrixSign_Mux/1(MatrixSign_33)
  Ground_4/1 -> VISUMatrixSign_Mux/2(MatrixSign_34)

== system TSR_Scenario_002_cs/HighwayOverhang_21 ==
  note: Resources
      |
      | 1: InformationSign\MatrixSign_GreenArrow.png: MatrixSign GreenArrow
      | 2: InformationSign\TASS_logo.jpeg: TASS logo
      | 3: InformationSign\SEARCH_Logo.png: SEARCH Logo
      | 4: InformationSign\z274-50_black_full.png: z274-50 black full
      | 5: InformationSign\z274-51_black_full.png: z274-51 black full
      | 6: InformationSign\z274-52_black_full.png: z274-52 black full
      | 7: InformationSign\z274-53_black_full.png: z274-53 black full
      | 8: InformationSign\z274-54_black_full.png: z274-54 black full
      | 9: InformationSign\z274-55_black_full.png: z274-55 black full
      | 10: InformationSign\z274-56_black_full.png: z274-56 black full
      | 11: InformationSign\z274-57_black_full.png: z274-57 black full
      | 12: InformationSign\z274-58_black_full.png: z274-58 black full
      | 13: InformationSign\z274-59_black_full.png: z274-59 black full
      | 14: InformationSign\z274-60_black_full.png: z274-60 black full
      | 15: InformationSign\z274-61_black_full.png: z274-61 black full
      | 16: InformationSign\z274-62_black_full.png: z274-62 black full
      | 17: InformationSign\z274-63_black_full.png: z274-63 black full
  note: Resources
  Ground_{1..4} (4 alike) : Ground
  VISUMatrixSignLights_Mux : Subsystem  Tag=MuxMatrixSignLights
      settings: DeleteFcn=PreScanBlockDeleteFcn;
      mask Type: PreScan Mux Block:
  VISUMatrixSign_Mux : Subsystem  Tag=MuxMatrixSign
      settings: DeleteFcn=PreScanBlockDeleteFcn;
      mask Type: PreScan Mux Block:
  VISUMatrixSign_HighwayOverhang_21 : Outport  Tag=VisuMatrixSignOutport  SampleTime=1/100
  VISUMatrixSignLights_HighwayOverhang_21 : Outport  Tag=VisuMatrixSignLightsOutport  Port=2  SampleTime=1/100
  wires:
  VISUMatrixSign_Mux/1(MatrixSigns) -> VISUMatrixSign_HighwayOverhang_21/1
  VISUMatrixSignLights_Mux/1(MatrixSignLights) -> VISUMatrixSignLights_HighwayOverhang_21/1
  Ground_1/1 -> VISUMatrixSignLights_Mux/1(MatrixSign_11)
  Ground_2/1 -> VISUMatrixSignLights_Mux/2(MatrixSign_53)
  Ground_3/1 -> VISUMatrixSign_Mux/1(MatrixSign_11)
  Ground_4/1 -> VISUMatrixSign_Mux/2(MatrixSign_53)

== system TSR_Scenario_002_cs/HighwayOverhang_22 ==
  note: Resources
      |
      | 1: InformationSign\MatrixSign_GreenArrow.png: MatrixSign GreenArrow
      | 2: InformationSign\TASS_logo.jpeg: TASS logo
      | 3: InformationSign\SEARCH_Logo.png: SEARCH Logo
      | 4: InformationSign\z274-50_black_full.png: z274-50 black full
      | 5: InformationSign\z274-51_black_full.png: z274-51 black full
      | 6: InformationSign\z274-52_black_full.png: z274-52 black full
      | 7: InformationSign\z274-53_black_full.png: z274-53 black full
      | 8: InformationSign\z274-54_black_full.png: z274-54 black full
      | 9: InformationSign\z274-55_black_full.png: z274-55 black full
      | 10: InformationSign\z274-56_black_full.png: z274-56 black full
      | 11: InformationSign\z274-57_black_full.png: z274-57 black full
      | 12: InformationSign\z274-58_black_full.png: z274-58 black full
      | 13: InformationSign\z274-59_black_full.png: z274-59 black full
      | 14: InformationSign\z274-60_black_full.png: z274-60 black full
      | 15: InformationSign\z274-61_black_full.png: z274-61 black full
      | 16: InformationSign\z274-62_black_full.png: z274-62 black full
      | 17: InformationSign\z274-63_black_full.png: z274-63 black full
  note: Resources
  Ground_{1..4} (4 alike) : Ground
  VISUMatrixSignLights_Mux : Subsystem  Tag=MuxMatrixSignLights
      settings: DeleteFcn=PreScanBlockDeleteFcn;
      mask Type: PreScan Mux Block:
  VISUMatrixSign_Mux : Subsystem  Tag=MuxMatrixSign
      settings: DeleteFcn=PreScanBlockDeleteFcn;
      mask Type: PreScan Mux Block:
  VISUMatrixSign_HighwayOverhang_22 : Outport  Tag=VisuMatrixSignOutport  SampleTime=1/100
  VISUMatrixSignLights_HighwayOverhang_22 : Outport  Tag=VisuMatrixSignLightsOutport  Port=2  SampleTime=1/100
  wires:
  VISUMatrixSign_Mux/1(MatrixSigns) -> VISUMatrixSign_HighwayOverhang_22/1
  VISUMatrixSignLights_Mux/1(MatrixSignLights) -> VISUMatrixSignLights_HighwayOverhang_22/1
  Ground_1/1 -> VISUMatrixSignLights_Mux/1(MatrixSign_35)
  Ground_2/1 -> VISUMatrixSignLights_Mux/2(MatrixSign_36)
  Ground_3/1 -> VISUMatrixSign_Mux/1(MatrixSign_35)
  Ground_4/1 -> VISUMatrixSign_Mux/2(MatrixSign_36)

== system TSR_Scenario_002_cs/HighwayOverhang_23 ==
  note: Resources
      |
      | 1: InformationSign\MatrixSign_GreenArrow.png: MatrixSign GreenArrow
      | 2: InformationSign\TASS_logo.jpeg: TASS logo
      | 3: InformationSign\SEARCH_Logo.png: SEARCH Logo
      | 4: InformationSign\z274-50_black_full.png: z274-50 black full
      | 5: InformationSign\z274-51_black_full.png: z274-51 black full
      | 6: InformationSign\z274-52_black_full.png: z274-52 black full
      | 7: InformationSign\z274-53_black_full.png: z274-53 black full
      | 8: InformationSign\z274-54_black_full.png: z274-54 black full
      | 9: InformationSign\z274-55_black_full.png: z274-55 black full
      | 10: InformationSign\z274-56_black_full.png: z274-56 black full
      | 11: InformationSign\z274-57_black_full.png: z274-57 black full
      | 12: InformationSign\z274-58_black_full.png: z274-58 black full
      | 13: InformationSign\z274-59_black_full.png: z274-59 black full
      | 14: InformationSign\z274-60_black_full.png: z274-60 black full
      | 15: InformationSign\z274-61_black_full.png: z274-61 black full
      | 16: InformationSign\z274-62_black_full.png: z274-62 black full
      | 17: InformationSign\z274-63_black_full.png: z274-63 black full
  note: Resources
  Ground_{1..4} (4 alike) : Ground
  VISUMatrixSignLights_Mux : Subsystem  Tag=MuxMatrixSignLights
      settings: DeleteFcn=PreScanBlockDeleteFcn;
      mask Type: PreScan Mux Block:
  VISUMatrixSign_Mux : Subsystem  Tag=MuxMatrixSign
      settings: DeleteFcn=PreScanBlockDeleteFcn;
      mask Type: PreScan Mux Block:
  VISUMatrixSign_HighwayOverhang_23 : Outport  Tag=VisuMatrixSignOutport  SampleTime=1/100
  VISUMatrixSignLights_HighwayOverhang_23 : Outport  Tag=VisuMatrixSignLightsOutport  Port=2  SampleTime=1/100
  wires:
  VISUMatrixSign_Mux/1(MatrixSigns) -> VISUMatrixSign_HighwayOverhang_23/1
  VISUMatrixSignLights_Mux/1(MatrixSignLights) -> VISUMatrixSignLights_HighwayOverhang_23/1
  Ground_1/1 -> VISUMatrixSignLights_Mux/1(MatrixSign_13)
  Ground_2/1 -> VISUMatrixSignLights_Mux/2(MatrixSign_54)
  Ground_3/1 -> VISUMatrixSign_Mux/1(MatrixSign_13)
  Ground_4/1 -> VISUMatrixSign_Mux/2(MatrixSign_54)

== system TSR_Scenario_002_cs/HighwayOverhang_24 ==
  note: Resources
      |
      | 1: InformationSign\MatrixSign_GreenArrow.png: MatrixSign GreenArrow
      | 2: InformationSign\TASS_logo.jpeg: TASS logo
      | 3: InformationSign\SEARCH_Logo.png: SEARCH Logo
      | 4: InformationSign\z274-50_black_full.png: z274-50 black full
      | 5: InformationSign\z274-51_black_full.png: z274-51 black full
      | 6: InformationSign\z274-52_black_full.png: z274-52 black full
      | 7: InformationSign\z274-53_black_full.png: z274-53 black full
      | 8: InformationSign\z274-54_black_full.png: z274-54 black full
      | 9: InformationSign\z274-55_black_full.png: z274-55 black full
      | 10: InformationSign\z274-56_black_full.png: z274-56 black full
      | 11: InformationSign\z274-57_black_full.png: z274-57 black full
      | 12: InformationSign\z274-58_black_full.png: z274-58 black full
      | 13: InformationSign\z274-59_black_full.png: z274-59 black full
      | 14: InformationSign\z274-60_black_full.png: z274-60 black full
      | 15: InformationSign\z274-61_black_full.png: z274-61 black full
      | 16: InformationSign\z274-62_black_full.png: z274-62 black full
      | 17: InformationSign\z274-63_black_full.png: z274-63 black full
  note: Resources
  Ground_{1..4} (4 alike) : Ground
  VISUMatrixSignLights_Mux : Subsystem  Tag=MuxMatrixSignLights
      settings: DeleteFcn=PreScanBlockDeleteFcn;
      mask Type: PreScan Mux Block:
  VISUMatrixSign_Mux : Subsystem  Tag=MuxMatrixSign
      settings: DeleteFcn=PreScanBlockDeleteFcn;
      mask Type: PreScan Mux Block:
  VISUMatrixSign_HighwayOverhang_24 : Outport  Tag=VisuMatrixSignOutport  SampleTime=1/100
  VISUMatrixSignLights_HighwayOverhang_24 : Outport  Tag=VisuMatrixSignLightsOutport  Port=2  SampleTime=1/100
  wires:
  VISUMatrixSign_Mux/1(MatrixSigns) -> VISUMatrixSign_HighwayOverhang_24/1
  VISUMatrixSignLights_Mux/1(MatrixSignLights) -> VISUMatrixSignLights_HighwayOverhang_24/1
  Ground_1/1 -> VISUMatrixSignLights_Mux/1(MatrixSign_25)
  Ground_2/1 -> VISUMatrixSignLights_Mux/2(MatrixSign_55)
  Ground_3/1 -> VISUMatrixSign_Mux/1(MatrixSign_25)
  Ground_4/1 -> VISUMatrixSign_Mux/2(MatrixSign_55)

== system TSR_Scenario_002_cs/HighwayOverhang_25 ==
  note: Resources
      |
      | 1: InformationSign\MatrixSign_GreenArrow.png: MatrixSign GreenArrow
      | 2: InformationSign\TASS_logo.jpeg: TASS logo
      | 3: InformationSign\SEARCH_Logo.png: SEARCH Logo
      | 4: InformationSign\z274-50_black_full.png: z274-50 black full
      | 5: InformationSign\z274-51_black_full.png: z274-51 black full
      | 6: InformationSign\z274-52_black_full.png: z274-52 black full
      | 7: InformationSign\z274-53_black_full.png: z274-53 black full
      | 8: InformationSign\z274-54_black_full.png: z274-54 black full
      | 9: InformationSign\z274-55_black_full.png: z274-55 black full
      | 10: InformationSign\z274-56_black_full.png: z274-56 black full
      | 11: InformationSign\z274-57_black_full.png: z274-57 black full
      | 12: InformationSign\z274-58_black_full.png: z274-58 black full
      | 13: InformationSign\z274-59_black_full.png: z274-59 black full
      | 14: InformationSign\z274-60_black_full.png: z274-60 black full
      | 15: InformationSign\z274-61_black_full.png: z274-61 black full
      | 16: InformationSign\z274-62_black_full.png: z274-62 black full
      | 17: InformationSign\z274-63_black_full.png: z274-63 black full
  note: Resources
  Ground_{1..4} (4 alike) : Ground
  VISUMatrixSignLights_Mux : Subsystem  Tag=MuxMatrixSignLights
      settings: DeleteFcn=PreScanBlockDeleteFcn;
      mask Type: PreScan Mux Block:
  VISUMatrixSign_Mux : Subsystem  Tag=MuxMatrixSign
      settings: DeleteFcn=PreScanBlockDeleteFcn;
      mask Type: PreScan Mux Block:
  VISUMatrixSign_HighwayOverhang_25 : Outport  Tag=VisuMatrixSignOutport  SampleTime=1/100
  VISUMatrixSignLights_HighwayOverhang_25 : Outport  Tag=VisuMatrixSignLightsOutport  Port=2  SampleTime=1/100
  wires:
  VISUMatrixSign_Mux/1(MatrixSigns) -> VISUMatrixSign_HighwayOverhang_25/1
  VISUMatrixSignLights_Mux/1(MatrixSignLights) -> VISUMatrixSignLights_HighwayOverhang_25/1
  Ground_1/1 -> VISUMatrixSignLights_Mux/1(MatrixSign_26)
  Ground_2/1 -> VISUMatrixSignLights_Mux/2(MatrixSign_56)
  Ground_3/1 -> VISUMatrixSign_Mux/1(MatrixSign_26)
  Ground_4/1 -> VISUMatrixSign_Mux/2(MatrixSign_56)

== system TSR_Scenario_002_cs/HighwayOverhang_26 ==
  note: Resources
      |
      | 1: InformationSign\MatrixSign_GreenArrow.png: MatrixSign GreenArrow
      | 2: InformationSign\TASS_logo.jpeg: TASS logo
      | 3: InformationSign\SEARCH_Logo.png: SEARCH Logo
      | 4: InformationSign\z274-50_black_full.png: z274-50 black full
      | 5: InformationSign\z274-51_black_full.png: z274-51 black full
      | 6: InformationSign\z274-52_black_full.png: z274-52 black full
      | 7: InformationSign\z274-53_black_full.png: z274-53 black full
      | 8: InformationSign\z274-54_black_full.png: z274-54 black full
      | 9: InformationSign\z274-55_black_full.png: z274-55 black full
      | 10: InformationSign\z274-56_black_full.png: z274-56 black full
      | 11: InformationSign\z274-57_black_full.png: z274-57 black full
      | 12: InformationSign\z274-58_black_full.png: z274-58 black full
      | 13: InformationSign\z274-59_black_full.png: z274-59 black full
      | 14: InformationSign\z274-60_black_full.png: z274-60 black full
      | 15: InformationSign\z274-61_black_full.png: z274-61 black full
      | 16: InformationSign\z274-62_black_full.png: z274-62 black full
      | 17: InformationSign\z274-63_black_full.png: z274-63 black full
  note: Resources
  Ground_{1..4} (4 alike) : Ground
  VISUMatrixSignLights_Mux : Subsystem  Tag=MuxMatrixSignLights
      settings: DeleteFcn=PreScanBlockDeleteFcn;
      mask Type: PreScan Mux Block:
  VISUMatrixSign_Mux : Subsystem  Tag=MuxMatrixSign
      settings: DeleteFcn=PreScanBlockDeleteFcn;
      mask Type: PreScan Mux Block:
  VISUMatrixSign_HighwayOverhang_26 : Outport  Tag=VisuMatrixSignOutport  SampleTime=1/100
  VISUMatrixSignLights_HighwayOverhang_26 : Outport  Tag=VisuMatrixSignLightsOutport  Port=2  SampleTime=1/100
  wires:
  VISUMatrixSign_Mux/1(MatrixSigns) -> VISUMatrixSign_HighwayOverhang_26/1
  VISUMatrixSignLights_Mux/1(MatrixSignLights) -> VISUMatrixSignLights_HighwayOverhang_26/1
  Ground_1/1 -> VISUMatrixSignLights_Mux/1(MatrixSign_37)
  Ground_2/1 -> VISUMatrixSignLights_Mux/2(MatrixSign_38)
  Ground_3/1 -> VISUMatrixSign_Mux/1(MatrixSign_37)
  Ground_4/1 -> VISUMatrixSign_Mux/2(MatrixSign_38)

== system TSR_Scenario_002_cs/HighwayOverhang_27 ==
  note: Resources
      |
      | 1: InformationSign\MatrixSign_GreenArrow.png: MatrixSign GreenArrow
      | 2: InformationSign\TASS_logo.jpeg: TASS logo
      | 3: InformationSign\SEARCH_Logo.png: SEARCH Logo
      | 4: InformationSign\z274-50_black_full.png: z274-50 black full
      | 5: InformationSign\z274-51_black_full.png: z274-51 black full
      | 6: InformationSign\z274-52_black_full.png: z274-52 black full
      | 7: InformationSign\z274-53_black_full.png: z274-53 black full
      | 8: InformationSign\z274-54_black_full.png: z274-54 black full
      | 9: InformationSign\z274-55_black_full.png: z274-55 black full
      | 10: InformationSign\z274-56_black_full.png: z274-56 black full
      | 11: InformationSign\z274-57_black_full.png: z274-57 black full
      | 12: InformationSign\z274-58_black_full.png: z274-58 black full
      | 13: InformationSign\z274-59_black_full.png: z274-59 black full
      | 14: InformationSign\z274-60_black_full.png: z274-60 black full
      | 15: InformationSign\z274-61_black_full.png: z274-61 black full
      | 16: InformationSign\z274-62_black_full.png: z274-62 black full
      | 17: InformationSign\z274-63_black_full.png: z274-63 black full
  note: Resources
  Ground_{1..4} (4 alike) : Ground
  VISUMatrixSignLights_Mux : Subsystem  Tag=MuxMatrixSignLights
      settings: DeleteFcn=PreScanBlockDeleteFcn;
      mask Type: PreScan Mux Block:
  VISUMatrixSign_Mux : Subsystem  Tag=MuxMatrixSign
      settings: DeleteFcn=PreScanBlockDeleteFcn;
      mask Type: PreScan Mux Block:
  VISUMatrixSign_HighwayOverhang_27 : Outport  Tag=VisuMatrixSignOutport  SampleTime=1/100
  VISUMatrixSignLights_HighwayOverhang_27 : Outport  Tag=VisuMatrixSignLightsOutport  Port=2  SampleTime=1/100
  wires:
  VISUMatrixSign_Mux/1(MatrixSigns) -> VISUMatrixSign_HighwayOverhang_27/1
  VISUMatrixSignLights_Mux/1(MatrixSignLights) -> VISUMatrixSignLights_HighwayOverhang_27/1
  Ground_1/1 -> VISUMatrixSignLights_Mux/1(MatrixSign_39)
  Ground_2/1 -> VISUMatrixSignLights_Mux/2(MatrixSign_40)
  Ground_3/1 -> VISUMatrixSign_Mux/1(MatrixSign_39)
  Ground_4/1 -> VISUMatrixSign_Mux/2(MatrixSign_40)

== system TSR_Scenario_002_cs/HighwayOverhang_28 ==
  note: Resources
      |
      | 1: InformationSign\MatrixSign_GreenArrow.png: MatrixSign GreenArrow
      | 2: InformationSign\TASS_logo.jpeg: TASS logo
      | 3: InformationSign\SEARCH_Logo.png: SEARCH Logo
      | 4: InformationSign\z274-50_black_full.png: z274-50 black full
      | 5: InformationSign\z274-51_black_full.png: z274-51 black full
      | 6: InformationSign\z274-52_black_full.png: z274-52 black full
      | 7: InformationSign\z274-53_black_full.png: z274-53 black full
      | 8: InformationSign\z274-54_black_full.png: z274-54 black full
      | 9: InformationSign\z274-55_black_full.png: z274-55 black full
      | 10: InformationSign\z274-56_black_full.png: z274-56 black full
      | 11: InformationSign\z274-57_black_full.png: z274-57 black full
      | 12: InformationSign\z274-58_black_full.png: z274-58 black full
      | 13: InformationSign\z274-59_black_full.png: z274-59 black full
      | 14: InformationSign\z274-60_black_full.png: z274-60 black full
      | 15: InformationSign\z274-61_black_full.png: z274-61 black full
      | 16: InformationSign\z274-62_black_full.png: z274-62 black full
      | 17: InformationSign\z274-63_black_full.png: z274-63 black full
  note: Resources
  Ground_{1..4} (4 alike) : Ground
  VISUMatrixSignLights_Mux : Subsystem  Tag=MuxMatrixSignLights
      settings: DeleteFcn=PreScanBlockDeleteFcn;
      mask Type: PreScan Mux Block:
  VISUMatrixSign_Mux : Subsystem  Tag=MuxMatrixSign
      settings: DeleteFcn=PreScanBlockDeleteFcn;
      mask Type: PreScan Mux Block:
  VISUMatrixSign_HighwayOverhang_28 : Outport  Tag=VisuMatrixSignOutport  SampleTime=1/100
  VISUMatrixSignLights_HighwayOverhang_28 : Outport  Tag=VisuMatrixSignLightsOutport  Port=2  SampleTime=1/100
  wires:
  VISUMatrixSign_Mux/1(MatrixSigns) -> VISUMatrixSign_HighwayOverhang_28/1
  VISUMatrixSignLights_Mux/1(MatrixSignLights) -> VISUMatrixSignLights_HighwayOverhang_28/1
  Ground_1/1 -> VISUMatrixSignLights_Mux/1(MatrixSign_41)
  Ground_2/1 -> VISUMatrixSignLights_Mux/2(MatrixSign_42)
  Ground_3/1 -> VISUMatrixSign_Mux/1(MatrixSign_41)
  Ground_4/1 -> VISUMatrixSign_Mux/2(MatrixSign_42)

== system TSR_Scenario_002_cs/HighwayOverhang_3 ==
  note: Resources
      |
      | 1: InformationSign\MatrixSign_GreenArrow.png: MatrixSign GreenArrow
      | 2: InformationSign\TASS_logo.jpeg: TASS logo
      | 3: InformationSign\SEARCH_Logo.png: SEARCH Logo
      | 4: InformationSign\z274-50_black_full.png: z274-50 black full
      | 5: InformationSign\z274-51_black_full.png: z274-51 black full
      | 6: InformationSign\z274-52_black_full.png: z274-52 black full
      | 7: InformationSign\z274-53_black_full.png: z274-53 black full
      | 8: InformationSign\z274-54_black_full.png: z274-54 black full
      | 9: InformationSign\z274-55_black_full.png: z274-55 black full
      | 10: InformationSign\z274-56_black_full.png: z274-56 black full
      | 11: InformationSign\z274-57_black_full.png: z274-57 black full
      | 12: InformationSign\z274-58_black_full.png: z274-58 black full
      | 13: InformationSign\z274-59_black_full.png: z274-59 black full
      | 14: InformationSign\z274-60_black_full.png: z274-60 black full
      | 15: InformationSign\z274-61_black_full.png: z274-61 black full
      | 16: InformationSign\z274-62_black_full.png: z274-62 black full
      | 17: InformationSign\z274-63_black_full.png: z274-63 black full
  note: Resources
  Ground_{1..4} (4 alike) : Ground
  VISUMatrixSignLights_Mux : Subsystem  Tag=MuxMatrixSignLights
      settings: DeleteFcn=PreScanBlockDeleteFcn;
      mask Type: PreScan Mux Block:
  VISUMatrixSign_Mux : Subsystem  Tag=MuxMatrixSign
      settings: DeleteFcn=PreScanBlockDeleteFcn;
      mask Type: PreScan Mux Block:
  VISUMatrixSign_HighwayOverhang_3 : Outport  Tag=VisuMatrixSignOutport  SampleTime=1/100
  VISUMatrixSignLights_HighwayOverhang_3 : Outport  Tag=VisuMatrixSignLightsOutport  Port=2  SampleTime=1/100
  wires:
  VISUMatrixSign_Mux/1(MatrixSigns) -> VISUMatrixSign_HighwayOverhang_3/1
  VISUMatrixSignLights_Mux/1(MatrixSignLights) -> VISUMatrixSignLights_HighwayOverhang_3/1
  Ground_1/1 -> VISUMatrixSignLights_Mux/1(MatrixSign_12)
  Ground_2/1 -> VISUMatrixSignLights_Mux/2(MatrixSign_50)
  Ground_3/1 -> VISUMatrixSign_Mux/1(MatrixSign_12)
  Ground_4/1 -> VISUMatrixSign_Mux/2(MatrixSign_50)
